simulink model "SdbTxSigProc"  (R2022b)
settings: Solver=FixedStepDiscrete  StartTime=0.0  StopTime=10.0  FixedStep=0.1  RelTol=1e-3
(part 2 of 4)

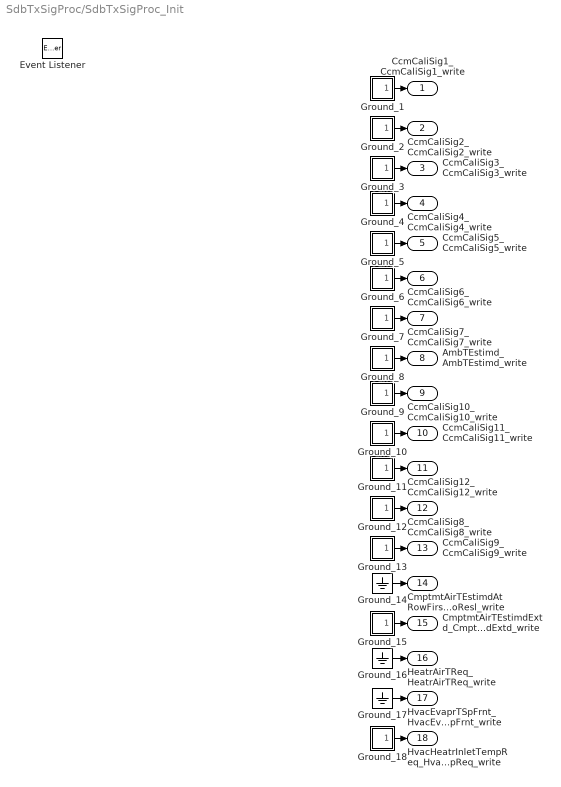
== system SdbTxSigProc/SdbTxSigProc_Init ==
  Event Listener : EventListener  EventName=reset
  Ground_1 : Subsystem
  Ground_10 : Subsystem
  Ground_11 : Subsystem
  Ground_12 : Subsystem
  Ground_13 : Subsystem
  Ground_14 : Ground
  Ground_15 : Subsystem
  Ground_16 : Ground
  Ground_17 : Ground
  Ground_18 : Subsystem
  Ground_2 : Subsystem
  Ground_3 : Subsystem
  Ground_4 : Subsystem
  Ground_5 : Subsystem
  Ground_6 : Subsystem
  Ground_7 : Subsystem
  Ground_8 : Subsystem
  Ground_9 : Subsystem
  CcmCaliSig1_CcmCaliSig1_write : Outport
  CcmCaliSig2_CcmCaliSig2_write : Outport  Port=2
  CcmCaliSig3_CcmCaliSig3_write : Outport  Port=3
  CcmCaliSig4_CcmCaliSig4_write : Outport  Port=4
  CcmCaliSig5_CcmCaliSig5_write : Outport  Port=5
  CcmCaliSig6_CcmCaliSig6_write : Outport  Port=6
  CcmCaliSig7_CcmCaliSig7_write : Outport  Port=7
  AmbTEstimd_AmbTEstimd_write : Outport  Port=8
  CcmCaliSig10_CcmCaliSig10_write : Outport  Port=9
  CcmCaliSig11_CcmCaliSig11_write : Outport  Port=10
  CcmCaliSig12_CcmCaliSig12_write : Outport  Port=11
  CcmCaliSig8_CcmCaliSig8_write : Outport  Port=12
  CcmCaliSig9_CcmCaliSig9_write : Outport  Port=13
  CmptmtAirTEstimdAtRowFirstLoResl_CmptmtAirTEstimdAtRowFirstLoResl_write : Outport  Port=14
  CmptmtAirTEstimdExtd_CmptmtAirTEstimdExtd_write : Outport  Port=15
  HeatrAirTReq_HeatrAirTReq_write : Outport  Port=16
  HvacEvaprTSpFrnt_HvacEvaprTSpFrnt_write : Outport  Port=17
  HvacHeatrInletTempReq_HvacHeatrInletTempReq_write : Outport  Port=18
  wires:
  Ground_1/1(Out1) -> CcmCaliSig1_CcmCaliSig1_write/1
  Ground_2/1(Out1) -> CcmCaliSig2_CcmCaliSig2_write/1
  Ground_3/1(Out1) -> CcmCaliSig3_CcmCaliSig3_write/1
  Ground_4/1(Out1) -> CcmCaliSig4_CcmCaliSig4_write/1
  Ground_5/1(Out1) -> CcmCaliSig5_CcmCaliSig5_write/1
  Ground_6/1(Out1) -> CcmCaliSig6_CcmCaliSig6_write/1
  Ground_7/1(Out1) -> CcmCaliSig7_CcmCaliSig7_write/1
  Ground_8/1(Out1) -> AmbTEstimd_AmbTEstimd_write/1
  Ground_9/1(Out1) -> CcmCaliSig10_CcmCaliSig10_write/1
  Ground_10/1(Out1) -> CcmCaliSig11_CcmCaliSig11_write/1
  Ground_11/1(Out1) -> CcmCaliSig12_CcmCaliSig12_write/1
  Ground_12/1(Out1) -> CcmCaliSig8_CcmCaliSig8_write/1
  Ground_13/1(Out1) -> CcmCaliSig9_CcmCaliSig9_write/1
  Ground_14/1 -> CmptmtAirTEstimdAtRowFirstLoResl_CmptmtAirTEstimdAtRowFirstLoResl_write/1
  Ground_15/1(Out1) -> CmptmtAirTEstimdExtd_CmptmtAirTEstimdExtd_write/1
  Ground_16/1 -> HeatrAirTReq_HeatrAirTReq_write/1
  Ground_17/1 -> HvacEvaprTSpFrnt_HvacEvaprTSpFrnt_write/1
  Ground_18/1(Out1) -> HvacHeatrInletTempReq_HvacHeatrInletTempReq_write/1
